simulink model "BaseEngineController_A02_bak"  (Simulink 7.7)
settings: Solver=FixedStepDiscrete  StartTime=0.0  StopTime=10.0  RelTol=1e-3
PreLoadFcn:
  | try, eval([bdroot '_setup']); catch, eval('model_setup', ''); end
InitFcn:
  | try, eval([bdroot '_setup']); catch, eval('model_setup', ''); end
undefined symbols (not defined in the file or in its folder): ECU555, High, motohawk_build_version, motohawk_get_faults, motohawk_trunc_name, motohawk_vardec_config_group, motohawk_vardec_path, True
libraries: MotoHawk_lib x1145, AutoDoc_lib x786, motohawk_control_primitives_lib x114, motohawk_engine_lib x19, motohawk_annotations_lib x12, simulink x11, simulink_extras x1
(part 1 of 23)
Description:
  | MI07 SECM48
CloseFcn:
  | rtwprivate ssgencode ModelCloseRequest BaseEngineController_A02
settings Model: MotoHawkBuildVersion=116  MotoHawkBuildStats=struct('TotalFLASH',{373552},'TotalEEPROM',{1254},'TotalRAM',{26040},'AppFLASH',{154160},'AppEEPROM',{1080},'AppRAM',{7766},'HeapRAM',{4096},'BuildSuccessCount',{8},'BuildErrorCount',{29},'OSRAM',{4962},'StackRAM',{9216},'FgndTimeStackSize',{4096},'FgndAngleStackSize',{1024},'BgndStackSize',{2048},'IdleStackSize',{1024},'InterruptStackSize',{1024},'RTWBlockIOSize',{2192},'RTWDWorkSize',{1776},'RTWPrevZCSize',{0},'VolatileSize',{1668},'NonVolatileSize',{1080},'ConstantSize',{18616},'NonVolatileFormatID',{32179})  MotoHawkModelSavedName=BaseEngineController_A02_bak  MotoHawkModelGUID=dbd4ef7b-9d85-4e51-bb-d0-da-ee-71-31-1c  MotoHawkModelOpenTime=2018 4 5 16 53 42.812  MotoHawkModelElapsedTime=-3947112212.687  MotoHawkBuildGUID=66e2ce16-351c-4ae6-a6-cb-00-88-ae-da-ff  MotoHawkBuildInfo=struct('model_name',{'BaseEngineController_A02'},'motohawk_version',{'2011a_sp0.184'},'motohawk_component_version',{'0.0.7'},'matlab_version',{'7.12.0'},'build_version',{'115'},'module_type',{'ECU555-80'},'layout_type',{'DEV'},'processor_family',{'PPC'},'processor_type',{'MPC555'},'toolchain_vendor',{'gcc-powerpc-eabi'},'toolchain_version',{'4.4.0'},'component',{0},'archive_file',{'PCM.a'},'enable_build',{1},'fptype',{0},'flash_size',{368640},'ee_size',{8192},'ram_size',{26624},'decram_size',{0},'module_memory_def',{'MPC555_ModMemoryDef_8KEE_Dev'},'eri_table0_rev',{9},'max_eri_table_size',{65535},'use_debug_vardecs',{1},'use_info_vardecs',{1},'fgnd_base_period_ms',{5},'bgnd_base_period_ms',{50},'root_build_dir',{'C:\Users\HYBRID\Documents\GitHub\Wisconsin-Hybrid\EngineController_Rev1'},'build_dir',{'C:\Users\HYBRID\Documents\GitHub\Wisconsin-Hybrid\EngineController_Rev1\BaseEngineController_A02_Build'},'dll_name',{'BaseEngin_115'},'srz_name',{'BaseEngineController_A02_115'},'fgnd_stack_size',{4096},'fgnd_angle_stack_size',{1024},'bgnd_stack_size',{2048},'idle_stack_size',{1024},'irq_stack_size',{1024},'heap_size',{4096},'heap_margin',{409},'install_dll_dir',{'C:\ECUFiles\TDBDLL\'},'install_srz_dir',{'C:\ECUFiles\Programs'},'app_int_stack_size',{1024},'shutdown_stack_size',{1024},'mingw_dir',{'C:\PROGRA~2\Woodward\DEVELO~1\TOOLCH~1\GCC\win32-pe\4_4_0\'},'motohawk_dir',{'C:\PROGRA~2\Woodward\MCS\MotoHawk\2011A_~1.184'},'toolchain_dir',{'C:\Program Files (x86)\Woodward\DevelopmentTools\Toolchains\GCC\powerpc-eabi\4_4_0\'},'matlab_root',{'C:\Program Files (x86)\MATLAB\R2011a'},'model_guid',{'34a85f28-21b7-4112-82-b0-87-11-cc-cb-8f'},'build_guid',{'66e2ce16-351c-4ae6-a6-cb-00-88-ae-da-ff'},'token_guid',{'DD8AD11F95A6FC42BFF9AC780840E4B4'})  RegisteredStructs=struct('Name',{'FreezeFrame','FreezeFrames'},'Data',{struct('Name',{int32(0)},'Code',{int32(0)},'Var1',{int32(0)},'Var2',{int32(0)},'Var3',{int32(0)},'Var4',{int32(0)},'Var5',{int32(0)},'Var6',{int32(0)},'Var7',{int32(0)},'Var8',{int32(0)},'Var9',{int32(0)},'Var10',{int32(0)}),struct('Name',{int32(0)},'Code',{int32(0)},'Var1',{int32(0)},'Var2',{int32(0)},'Var3',{int32(0)},'Var4',{int32(0)},'Var5',{int32(0)},'Var6',{int32(0)},'Var7',{int32(0)},'Var8',{int32(0)},'Var9',{int32(0)},'Var10',{int32(0)})},'Enums',{{{'None','APP_Sensors_Conflict','CamEdgesFault','CamSyncFault','CrankEdgesFault','CrankSyncFault','ECTOverTempFault','ECTRangeHigh','ECTRangeLow','ECT_IR_Fault','EST1_Open','EST1_Short','EST2_Open','EST2_Short','EST3_Open','EST3_Short','EST4_Open','EST4_Short','EST5_Open','EST5_Short','EST6_Open','EST6_Short','EST7_Open','EST7_Short','EST8_Open','EST8_Short','ETCSpringTest','ETC_Open_Fault','ETC_Sticking','FuelAdaptRangeHi','FuelAdaptRangeLow','FuelTempRangeHigh','FuelTempRangeLow','HBridgeFault_ETC','HardOverspeed','IATRangeHigh','IATRangeLow','IAT_IR_Fault','Inj1Open','Inj2Open','Inj3Open','Inj4Open','Inj5Open','Inj6Open','Inj7Open','Inj8Open','LSDFault_FuelPump','LSDFault_MIL','LSOFault_VVT','MAFRangeHigh','MAFRangeLow','MAPRangeHigh','MAPRangeLow','MAPTimeRangeHigh','MAPTimeRangeLow','MAP_IR_HI','MAP_IR_LO','MAP_STICKING','MediumOverspeed','O2FailedLean','O2FailedRich','O2NotActive','OilTempRangeHigh','OilTempRangeLow','SensVoltRangeHigh','SensVoltRangeLow','SoftOverspeed','SysVoltRangeHigh','SysVoltRangeLow','TPS_Sensors_Conflict'}},{{'None','CamEdgesFault','CamSyncFault','CrankEdgesFault','CrankSyncFault','ECTOverTempFault','ECTRangeHigh','ECTRangeLow','ECTSevereOverTempFault','EGRSenseRangeHigh','EGRSenseRangeLow','EST1_Open','EST1_Short','EST2_Open','EST2_Short','ETCSpringTest','ETC_Open_Fault','ETC_Sticking','FuelAdaptRangeHi','FuelAdaptRangeLow','FuelPresHigh_Fault','FuelPresLow_Fault','FuelPresRangeHigh','FuelPresRangeLow','FuelSensRangeHigh','FuelSensRangeLow','HBridgeFault_ETC','HardOverspeed','IATRangeHigh','IATRangeLow','Inj1Open','Inj2Open','LSDFault_FuelPump','LSDFault_OilPump','LSDFault_OxygenHeater','MAFRangeHigh','MAFRangeLow','MAPRangeHigh','MAPRangeLow','MAPTimeRangeHigh','MAPTimeRangeLow','MAP_IR_HI','MAP_IR_LO','MAP_STICKING','MediumOverspeed','NoOil_Fault','O2FailedLean','O2FailedRich','O2NotActive','O2_PostCatRangeHigh','O2_PostCatRangeLow','OilPresRangeHigh','OilPresRangeLow','SensVoltRangeHigh','SensVoltRangeLow','SoftOverspeed','SysVoltLow_Fault','SysVoltRangeHigh','SysVoltRangeLow','TPS1AdaptHiMax','TPS1AdaptHiMin','TPS1AdaptLoMax','TPS1AdaptLoMin','TPS1RangeHigh','TPS1RangeLow','TPS2AdaptHiMax','TPS2AdaptHiMin','TPS2AdaptLoMax','TPS2AdaptLoMin','TPS2RangeHigh','TPS2RangeLow','TPS_Sensors_Conflict'}}})  Creator=dbocci  LastModifiedBy=HYBRID
settings OptimizationCC: InlineParams=on  InitFltsAndDblsToZero=on  OptimizeModelRefInitCode=on  LifeSpan=1
settings DebuggingCC: SolverPrmCheckMsg=none  InheritedTsInSrcMsg=none  TasksWithSamePriorityMsg=none  StrictBusMsg=Warning
settings HardwareCC: ProdEndianess=BigEndian  ProdHWDeviceType=Specified
settings RTWCC: SystemTargetFile=motohawk_ert_rtw.tlc  TLCOptions="-aCustomNonFinites=\"motohawk_genrtnonfinite.tlc\""  MakeCommand=motohawk_make_rtw  TemplateMakefile=motohawk_ert_rtw.tmf  Description=MotoHawk(tm) Rapid Prototyping  ProcessScript=motohawk_ert_rtw_make_rtw_hook
settings CodeAppCC: IgnoreCustomStorageClasses=off  MaxIdLength=100  CustomSymbolStrField=$H$N$M
settings STFCustomTargetCC: FunctionExecutionProfile=off  ModelReferenceCompliant=off  CombineOutputUpdateFcns=on  ERTCustomFileBanners=on  SupportContinuousTime=off  SupportNonInlinedSFcns=off  SystemTargetFile=motohawk_ert_rtw.tlc
settings ERTTargetCC: FunctionExecutionProfile=off  MultiwordLength=2048  ModelReferenceCompliant=off  ParMdlRefBuildCompliant=off  CompOptLevelCompliant=off  ERTFirstTimeCompliant=off  ModelStepFunctionPrototypeControlCompliant=off  CPPClassGenCompliant=off  ERTCustomFileTemplate=example_file_process.tlc  ConvertIfToSwitch=off

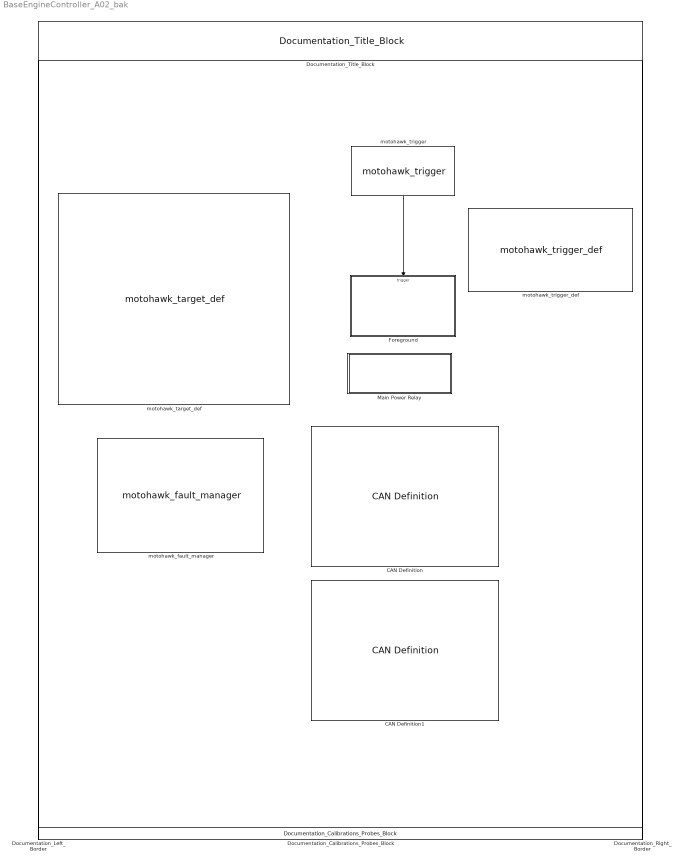
== system BaseEngineController_A02_bak ==
  CAN Definition : MotoHawk_lib/CAN Blocks/CAN Definition  Priority=-100  nam=['CAN_' num2str(1)]  text_resource=CAN1  code_resource=NONE  val_resource=-1  target=ECU555-80  bus=-2  btime=500 kbaud  pre=1  prop=0  pseg1=0  pseg2=0  resyn=0  tx_queue_size=16  rx_queue_size=16  use_protocol=on  city_id=hex2dec('B')  access_level=4  mototune_group='System | System Config | Communication Config'
  CAN Definition1 : MotoHawk_lib/CAN Blocks/CAN Definition  Priority=-100  nam=['CAN_' num2str(2)]  text_resource=CAN2  code_resource=NONE  val_resource=-1  target=ECU555-80  bus=-2  btime=500 kbaud  pre=1  prop=0  pseg1=0  pseg2=0  resyn=0  tx_queue_size=16  rx_queue_size=16  use_protocol=on  city_id=hex2dec('B')  access_level=4  mototune_group='System | System Config | Communication Config'
  Documentation_Calibrations_Probes_Block : AutoDoc_lib/Documentation_Calibrations_Probes_Block
  Documentation_Left_Border : AutoDoc_lib/Documentation_Left_Border  layout=Portrait
  Documentation_Right_Border : AutoDoc_lib/Documentation_Right_Border  layout=Portrait
  Documentation_Title_Block : AutoDoc_lib/Documentation_Title_Block
  Foreground : Subsystem  TreatAsAtomicUnit=on
      settings: RTWSystemCode=Function  RTWFcnNameOpts=Use subsystem name  RTWFileNameOpts=Use subsystem name
  Main Power Relay : Subsystem  AncestorBlock=MotoHawk_lib/Extra Development Blocks/Main Power Relay  mask(MotoHawk Main Power Relay Control){on_delay_count=2, off_delay_count=5, group='System | Main Power Relay'}
      mask prompts: on_delay_count "On Delay Count"; off_delay_count "Off Delay Count"; group "MotoTune Group"
      mask help:
      | This block provides basic control of the main power relay, by following the ECUP voltage input pin.
      |
      | Regardless of where the block is placed in a design, the strategy will execute every 50ms with background priority.
      |
      | Note that this block is simply composed of other primitive MotoHawk blocks and may be used as a starting point for a more complicated strategy.
      |
      | On Delay:  Once the ECU power goes high, after this period, the Main Power Relay will be turned on.
      | Off Delay:  Once the ECU power goes low, after this period, the Main Power Relay will be turned off.
      |
      | Note that one tick before MPRD is turned off, non-volatile variables will be stored, and the Shutdown event will be posted.  It is not necessary to use the Shutdown event.
      |
      | To delay shutdown based on Foreground events, use a data storage write block 'ShutDownTasksComplete' set as a boolean in the Foreground controller to initiate actual shutdown following completion of the tasks.
      |
      | ---------------------------------------------------------------------
      | Copyright 2005 MotoTron, Inc.  All Rights Reserved.
      mask init:
      | on_delay_lbl = sprintf('\nOn Delay: %d ms', on_delay_count * 50);
      | off_delay_lbl = sprintf('\nOff Delay: %d ms', off_delay_count * 50);
      mask Help: eval('motohawk_block_help(get_param(gcb,''MaskType''))');
      mask Display:
      | patch([0 0 1 1], [0 1 1 0], [220/255 220/255 220/255])
      | patch([0 0 1 1], [0 0.075 0.075 0], [230/255 0/255 0/255])
      | patch([0 0 1 1], [0.025 0.05 0.05 0.025], [150/255 0/255 0/255])
      | patch([0 0 1 1], [0 0.025 0.025 0], [0/255 0/255 0/255])
      | 
      | disp(['Main Power Relay' on_delay_lbl off_delay_lbl]);
  motohawk_fault_manager : MotoHawk_lib/Fault Management Blocks/motohawk_fault_manager  storage=FLASH  read_level=1  write_level=1  clear_level=1  xytype=uint16  mototune_group='Diagnostics & Protection | Faults'
  motohawk_target_def : MotoHawk_lib/motohawk_target_def  Priority=-65535  target=ECU555-80  layout=DEV  fptype=single (32 bits)  fgnd_stack_size=4*1024  fgnd_angle_stack_size=1*1024  bgnd_stack_size=2*1024  idle_stack_size=1*1024  irq_stack_size=1*1024  app_int_stack_size=1024  shutdown_stack_size=1024  heap_size=4*1024  show_margins=off  fgnd_stack_margin=(3*1024) * 0.1  fgnd_angle_stack_margin=0  bgnd_stack_margin=(2*1024) * 0.1  idle_stack_margin=(1024) * 0.1  irq_stack_margin=(1536) * 0.1  app_int_stack_margin=(1024) * 0.1  shutdown_stack_margin=(1024) * 0.1  heap_margin=(4*1024) * 0.1  comp_name=bdroot  comp_img='mh.jpg'  comp_security=No Encryption  comp_build_pass='mhbuild'  comp_sim_pass='mhsim'  comp_multi=off  show_filenames=on  dll_name=motohawk_trunc_name(bdroot, ['_' motohawk_build_version], 13)  srz_name=motohawk_trunc_name(bdroot, ['_' motohawk_build_version], 50)  build_dir=''  comp_dir='.'
  motohawk_trigger : MotoHawk_lib/Trigger Blocks/motohawk_trigger  Tag=Trigger  Priority=0  trigger=FGND_RTI_PERIODIC  function_name=Trigger_FGND_RTI_PERIODIC_10578p0002  priority_order=0  inherited=off  run_disabled=off  override_app_monitor=off  target=ECU555_80
  motohawk_trigger_def : MotoHawk_lib/Trigger Blocks/motohawk_trigger_def  fgnd_rti_rate=5000  bgnd_base_multiple=10  read_level=1  write_level=1  mototune_group='System | System Config | Trigger Config'
  wires:
  motohawk_trigger/1 -> Foreground/Trigger

== system BaseEngineController_A02_bak/Foreground ==
  f : TriggerPort  TriggerType=function-call
  Control : Subsystem  mask(Display Image File){image_file='pcm555.jpg'}
      mask prompts: image_file "Image File"
      mask help:
      | Enter an image file on the current MATLAB path to display on this block.
      |
      | Double-clicking this block opens the contents of the subsystem.  Right-click and select 'Mask parameters...' to select a new image file.
      |
      | To use just the mask of this block in a model, but supply your own contents, break the link to the library.
      mask init:
      | try,
      | 	img = imread(image_file);
      | 	motohawk_block_image(gcb, image_file);
      | catch,
      | 	img = imread('image_not_found.jpg');
      | end
      settings: OpenFcn=open_system(gcb,'force')  MoveFcn=motohawk_block_image(gcb, motohawk_get_param_ws(gcb, 'image_file'));
      mask Display: image(img);
  Documentation_Calibrations_Probes_Block : AutoDoc_lib/Documentation_Calibrations_Probes_Block
  Documentation_Left_Border : AutoDoc_lib/Documentation_Left_Border  layout=Portrait
  Documentation_Right_Border : AutoDoc_lib/Documentation_Right_Border  layout=Portrait
  Documentation_Title_Block : AutoDoc_lib/Documentation_Title_Block
  Inputs : Subsystem  mask(Display Image File){image_file='sensor.jpg'}
      mask prompts: image_file "Image File"
      mask help:
      | Enter an image file on the current MATLAB path to display on this block.
      |
      | Double-clicking this block opens the contents of the subsystem.  Right-click and select 'Mask parameters...' to select a new image file.
      |
      | To use just the mask of this block in a model, but supply your own contents, break the link to the library.
      mask init:
      | try,
      | 	img = imread(image_file);
      | 	motohawk_block_image(gcb, image_file);
      | catch,
      | 	img = imread('image_not_found.jpg');
      | end
      settings: OpenFcn=open_system(gcb,'force')  MoveFcn=motohawk_block_image(gcb, motohawk_get_param_ws(gcb, 'image_file'));
      mask Display: image(img);
  Outputs : Subsystem  mask(Display Image File){image_file='actuator.jpg'}
      mask prompts: image_file "Image File"
      mask help:
      | Enter an image file on the current MATLAB path to display on this block.
      |
      | Double-clicking this block opens the contents of the subsystem.  Right-click and select 'Mask parameters...' to select a new image file.
      |
      | To use just the mask of this block in a model, but supply your own contents, break the link to the library.
      mask init:
      | try,
      | 	img = imread(image_file);
      | 	motohawk_block_image(gcb, image_file);
      | catch,
      | 	img = imread('image_not_found.jpg');
      | end
      settings: OpenFcn=open_system(gcb,'force')  MoveFcn=motohawk_block_image(gcb, motohawk_get_param_ws(gcb, 'image_file'));
      mask Display: image(img);
  wires:
  Control/1(Actuators) -> Outputs/1(Actuators)
  Inputs/1(SensorBus) -> Control/1(Sensors)

== system BaseEngineController_A02_bak/Main Power Relay ==
  note: The saving and restoring of non-volatile variables
      | must be called from a background priority task.
  note: Always execute Main Power Relay control in the background.
  Documentation_Calibrations_Probes_Block : AutoDoc_lib/Documentation_Calibrations_Probes_Block
  Documentation_Left_Border : AutoDoc_lib/Documentation_Left_Border  layout=Portrait
  Documentation_Right_Border : AutoDoc_lib/Documentation_Right_Border  layout=Portrait
  Documentation_Title_Block : AutoDoc_lib/Documentation_Title_Block
  Main Power Relay : Subsystem  TreatAsAtomicUnit=on
  motohawk_trigger : MotoHawk_lib/Trigger Blocks/motohawk_trigger  Tag=Trigger  Priority=255  trigger=BGND_BASE_PERIODIC  function_name=Trigger_BGND_BASE_PERIODIC_9474p0004  priority_order=255  inherited=on  run_disabled=on  override_app_monitor=off  target=ECU555_80
  wires:
  motohawk_trigger/1 -> Main Power Relay/Trigger

== system BaseEngineController_A02_bak/Foreground/Control ==
  Sensors : Inport
  ActuatorCharacterization : Subsystem
  Controller : Subsystem
  Diagnostics : Subsystem  TreatAsAtomicUnit=on
  Documentation_Calibrations_Probes_Block : AutoDoc_lib/Documentation_Calibrations_Probes_Block
  Documentation_Left_Border : AutoDoc_lib/Documentation_Left_Border  layout=Portrait
  Documentation_Right_Border : AutoDoc_lib/Documentation_Right_Border  layout=Portrait
  Documentation_Title_Block : AutoDoc_lib/Documentation_Title_Block
  VirtualSensors : Subsystem
      settings: RTWSystemCode=Function  RTWFcnNameOpts=Use subsystem name  RTWFileNameOpts=Use function name
  motohawk_function_collector : MotoHawk_lib/Extra Development Blocks/motohawk_function_collector  SystemSampleTime=-1  count=5
  Actuators : Outport
  wires:
  Sensors/1 -> VirtualSensors/1(Raw)
  VirtualSensors/1(Sensors) -> Controller/1(Sensors), ActuatorCharacterization/2(Sensors), Diagnostics/2(Sensors)
  ActuatorCharacterization/1(Actuators) -> Actuators/1
  Controller/1(Actuators) -> ActuatorCharacterization/1(Control), Diagnostics/1(Control)
  Diagnostics/1(Diagnostics) -> ActuatorCharacterization/3(Diagnsotics)
  motohawk_function_collector/1 -> Diagnostics/Trigger

== system BaseEngineController_A02_bak/Foreground/Inputs ==
  Bus Creator : BusCreator  Inputs=7
  CAN Inputs : Subsystem
  Digital : Subsystem
  Documentation_Calibrations_Probes_Block : AutoDoc_lib/Documentation_Calibrations_Probes_Block
  Documentation_Left_Border : AutoDoc_lib/Documentation_Left_Border  layout=Portrait
  Documentation_Right_Border : AutoDoc_lib/Documentation_Right_Border  layout=Portrait
  Documentation_Title_Block : AutoDoc_lib/Documentation_Title_Block
  EngineSpeed : Subsystem
  LinearSensors : Subsystem
  NonLinearSensors : Subsystem
  PotentiometerSensors : Subsystem
  SensorBus : Outport
  wires:
  Digital/1(SensorBus) -> Bus Creator/6
  PotentiometerSensors/1(SensorBus) -> Bus Creator/5
  NonLinearSensors/1(SensorBus) -> Bus Creator/4
  LinearSensors/1(SensorBus) -> Bus Creator/3
  EngineSpeed/2(RPM) -> Bus Creator/2  "RPM"
  EngineSpeed/1(RPMInst) -> Bus Creator/1  "RPMInst"
  Bus Creator/1 -> SensorBus/1
  CAN Inputs/1(Out1) -> Bus Creator/7  "CAN Inputs"

== system BaseEngineController_A02_bak/Foreground/Outputs ==
  Actuators : Inport
  Bus Selector1 : BusSelector  OutputSignals=Controller.FUELP,Sensors.Raw.Digital.ESTOP
  Bus Selector2 : BusSelector  OutputSignals=Controller.OILP
  Bus Selector3 : BusSelector  OutputSignals=ActuatorCharacterization.ETC,Sensors.RawIndexes.SysVolt09Idx,Sensors.Raw.Digital.ESTOP,Sensors.RawProcessed.TimeSinceStall
  Bus Selector4 : BusSelector  OutputSignals=Controller.SOI,ActuatorCharacterization.InjMPW,ActuatorCharacterization.InjEPWOffset,ActuatorCharacterization.FuelCutSeq,Controller.MakeUpEOI
  Bus Selector5 : BusSelector  OutputSignals=ActuatorCharacterization.SparkEnable,ActuatorCharacterization.SparkStartAngle,Controller.SparkAdv,ActuatorCharacterization.SparkCutSeq
  Bus Selector6 : BusSelector  OutputSignals=Controller.O2 Heater
  CAN Out : Subsystem
  Coil Control : Subsystem  TreatAsAtomicUnit=on
  Documentation_Calibrations_Probes_Block : AutoDoc_lib/Documentation_Calibrations_Probes_Block
  Documentation_Left_Border : AutoDoc_lib/Documentation_Left_Border  layout=Portrait
  Documentation_Right_Border : AutoDoc_lib/Documentation_Right_Border  layout=Portrait
  Documentation_Title_Block : AutoDoc_lib/Documentation_Title_Block
  EGR Out : Subsystem
  FuelPump : Subsystem
  HBridge : Subsystem
  Injector Control : Subsystem
  Logical Operator4 : Logic  Operator=NOT  AllPortsSameDT=off  OutDataTypeStr=boolean
  Logical Operator5 : Logic  AllPortsSameDT=off  OutDataTypeStr=boolean
  Logical Operator9 : Logic  Operator=OR  AllPortsSameDT=off  OutDataTypeStr=boolean
  OilPump : Subsystem
  Oxygen Heater : Subsystem
  Relational Operator3 : RelationalOperator  Operator=>  ZeroCross=off  InputSameDT=on
  motohawk_calibration3 : MotoHawk_lib/Calibration & Probing Blocks/motohawk_calibration  nam='ETC_CutPower_StallTime'  val=15  storage=Calibration  show_more=on  name_source=Use Parameter  data_type=Inherit from 'Default Value'  read_level=1  write_level=1  ovr_cals=on  view_as=Number  enum_struct={'Disabled', 'Enabled'}  vector_style=Wide Row  use_help_units=on  help='Cut power to ETC after stalled this long'  units='s'  use_header_enum=off  row_header_enum={}  col_header_enum={}  use_min_max=off  min_val=0  max_val=100  use_precision=on  precision='2.3'  gain=1  offset=0  exp=1  use_mototune_group=on  mototune_group=motohawk_vardec_path(gcb)
  motohawk_trigger : MotoHawk_lib/Trigger Blocks/motohawk_trigger  Tag=Trigger  trigger=FGND_5XRTI_PERIODIC  function_name=Trigger_FGND_5XRTI_PERIODIC_9202p0004  priority_order=1  inherited=on  run_disabled=off  override_app_monitor=off  target=ECU555_80
  wires:
  Bus Selector3/2 -> HBridge/2(SysVoltIdx)  "<SysVolt09Idx>"
  Bus Selector4/5 -> Injector Control/5(MakeUpEOI)  "<MakeUpEOI>"
  Bus Selector3/1 -> HBridge/1(ETCDuty Cycle)  "<ETC>"
  Bus Selector5/4 -> Coil Control/4(CutSequence)  "<SparkCutSeq>"
  Bus Selector4/4 -> Injector Control/4(CutSequence)  "<FuelCutSeq>"
  Bus Selector5/3 -> Coil Control/3(SparkAdvance)  "<SparkAdv>"
  Bus Selector5/2 -> Coil Control/2(SparkStartAngle)  "<SparkStartAngle>"
  Bus Selector4/3 -> Injector Control/3(InjEPWOffset)  "<InjEPWOffset>"
  Bus Selector4/2 -> Injector Control/2(InjMPW)  "<InjMPW>"
  Bus Selector5/1 -> Coil Control/1(SparkEnable)  "<SparkEnable>"
  Bus Selector4/1 -> Injector Control/1(SOI)  "<SOI>"
  Actuators/1 -> Bus Selector3/1, Bus Selector1/1, Bus Selector2/1, EGR Out/1(EGR), CAN Out/1(In1), Bus Selector6/1, Bus Selector5/1, Bus Selector4/1
  motohawk_trigger/1 -> Coil Control/Trigger
  Bus Selector1/2 -> Logical Operator4/1  "<ESTOP>"
  Logical Operator4/1 -> Logical Operator5/2
  Bus Selector1/1 -> Logical Operator5/1  "<FUELP>"
  Logical Operator5/1 -> FuelPump/1(State)
  Logical Operator9/1 -> HBridge/3(ETC_CutPower)
  Bus Selector3/3 -> Logical Operator9/1  "<ESTOP>"
  Relational Operator3/1 -> Logical Operator9/2
  Bus Selector3/4 -> Relational Operator3/1  "<TimeSinceStall>"
  motohawk_calibration3/1 -> Relational Operator3/2
  Bus Selector2/1 -> OilPump/1(State)  "<OILP>"
  Bus Selector6/1 -> Oxygen Heater/1(State)  "<O2 Heater>"

== system BaseEngineController_A02_bak/Main Power Relay/Main Power Relay ==
  note: Provide Data Store
      | Access to MPRD State
  note: Control Main Power Relay
  note: NonVolatile Memory Store/Restore
      | Hooks from MotoTune
  note: Software Reset
      | Hook from MotoTune
  note: When ProcessorReboot goes true, it will force a falling edge to perform a shutdown, save NV vars, shutdown power, and then cause a processor reset if still true
  note: After a delay, turn on or off Main Power Relay
  f : TriggerPort  TriggerType=function-call  SampleTime=-1  ZeroCross=off
  Display Variable Event Boolean : MotoHawk_lib/Extra Development Blocks/Display Variable Event Boolean  SystemSampleTime=-1  nam='ProcessorReboot'  level=1  enum_str={'Run', 'Reboot'}  help_str='Reboots the processor when set.'  group=group
  Display Variable Event Function-Call : MotoHawk_lib/Extra Development Blocks/Display Variable Event Function-Call  SystemSampleTime=-1  nam='RestoreNVFactoryDefaults'  level=1  enum_str={'Run', 'Restore To Defaults'}  help_str='Restores NonVolatile Memory to Factory Defaults'  group='System | NonVolatile Storage'
  Display Variable Event Function-Call1 : MotoHawk_lib/Extra Development Blocks/Display Variable Event Function-Call  SystemSampleTime=-1  nam='StoreNV'  level=1  enum_str={'Run', 'Store To EEPROM'}  help_str='Stores RAM-Shadowed memory to serial EEPROM'  group='System | NonVolatile Storage'
  Documentation_Calibrations_Probes_Block : AutoDoc_lib/Documentation_Calibrations_Probes_Block
  Documentation_Left_Border : AutoDoc_lib/Documentation_Left_Border  layout=Portrait
  Documentation_Right_Border : AutoDoc_lib/Documentation_Right_Border  layout=Portrait
  Documentation_Title_Block : AutoDoc_lib/Documentation_Title_Block
  ECUP Latch : Stateflow chart  TreatAsAtomicUnit=on
  Forced Shutdown Process : Subsystem
  Logical Operator : Logic
  Logical Operator1 : Logic  Operator=NOT
  On Off Delay : Subsystem
  Relational Operator : RelationalOperator  ZeroCross=off  InputSameDT=on
  ShutDown Processes : Subsystem
  Unit Delay3 : UnitDelay  SampleTime=-1
  Unit Delay4 : UnitDelay  SampleTime=-1
  Unit Delay5 : UnitDelay  SampleTime=-1
  motohawk_ain5 : MotoHawk_lib/Analog I//O Blocks/motohawk_ain  res_name=[]  text_resource=ECUP  code_resource=RES_ECUP  val_resource=-1  target=ECU555-80  resource_enum_str=(None)=-1,ECUP=53,DRVP=54,XDRP=55,AN1M=56,AN2M=57,AN3M=58,AN4M=59,AN5M=60,AN6M=61,AN7M=62,AN8M=63,AN9M=64,AN10M=65,AN11M=66,AN12M=67,AN13M=68,AN14M=69,AN15M=70,AN16M=71,AN17M=72,AN18M=73,AN19M=74,O2A=90,O2B=91,XDRP_B=92  altref=5V Reference  typ=uint16  num_adc_bits=10  use_stat=off  use_vardec=off  nam='AIN'  read_level=1  write_level=1  group='System | I/O Pins | Inputs Config'
  motohawk_calibration : MotoHawk_lib/Calibration & Probing Blocks/motohawk_calibration  nam='ECUP_Threshold'  val=100  storage=Calibration  show_more=on  name_source=Use Parameter  data_type=Inherit via back propagation  read_level=1  write_level=1  ovr_cals=on  view_as=Number  enum_struct={'Disabled', 'Enabled'}  vector_style=Wide Row  use_help_units=off  help='ADC voltage threshold above which ECU is considered powered'  units='ADC_counts'  use_header_enum=off  row_header_enum={}  col_header_enum={}  use_min_max=on  min_val=0  max_val=1023  use_precision=off  precision=''  gain=1  offset=0  exp=1  use_mototune_group=on  mototune_group=motohawk_vardec_config_group(group)
  motohawk_data_def : MotoHawk_lib/Data Storage Blocks/motohawk_data_def  nam='MPRD'  container_nam=''  data=false  typ=Inherit from 'Initial Value'  struct_nam='MyStruct'  storage=Volatile  outputRef=off  use_vardec=on  window=Display  read_level=1  write_level=(Read Only)  ovr_cals=on  view_as=Enumeration  enum_struct={'Disabled', 'Enabled'}  vector_style=Wide Row  use_help_units=on  help='Indicates whether the main power relay is active'  units=''  use_header_enum=off  row_header_enum={}  col_header_enum={}  use_min_max=off  min_val=-inf  max_val=inf  use_precision=off  precision=''  gain=1  offset=0  exp=1  use_mototune_group=on  mototune_group=group  eval_name=MPRD  data_stores=motohawk_sfun_data_def_data_stores  struct_instances=motohawk_sfun_data_def_struct_instances  vardecs=motohawk_sfun_data_def_vardecs
  motohawk_data_def1 : MotoHawk_lib/Data Storage Blocks/motohawk_data_def  nam='ShutDownTasksComplete'  container_nam=''  data=false  typ=boolean  struct_nam='MyStruct'  storage=Volatile  outputRef=off  use_vardec=on  window=Display  read_level=1  write_level=1  ovr_cals=on  view_as=Enumeration  enum_struct={'False', 'True'}  vector_style=Wide Row  use_help_units=off  help=''  units=''  use_header_enum=off  row_header_enum={}  col_header_enum={}  use_min_max=off  min_val=-inf  max_val=inf  use_precision=off  precision=''  gain=1  offset=0  exp=1  use_mototune_group=on  mototune_group='System | Main Power Relay'  eval_name=ShutDownTasksComplete  data_stores=motohawk_sfun_data_def_data_stores  struct_instances=motohawk_sfun_data_def_struct_instances  vardecs=motohawk_sfun_data_def_vardecs
  motohawk_data_read : MotoHawk_lib/Data Storage Blocks/motohawk_data_read  source=Lookup By Name  structure=Scalar  operation=Read entire data structure at once  sz_vec=4  sz_row=2  sz_col=3  typ=boolean  nam='ShutDownTasksComplete'  checkNull=on  struct_inst_name='Struct1'  struct_nam='MyStruct'
  motohawk_data_write : MotoHawk_lib/Data Storage Blocks/motohawk_data_write  source=Lookup By Name  structure=Scalar  operation=Write entire data structure at once  sz_vec=-1  sz_row=3  sz_col=2  typ=boolean  nam='MPRD'  checkNull=off  write_read_only=off  struct_inst_name='Struct1'  struct_name='MyStruct'
  motohawk_dout : MotoHawk_lib/Digital I//O Blocks/motohawk_dout  resource_name=[]  text_resource=MPRD  target=ECU555-80  use_diag=off  use_vardec=off  nam='DOUT'  read_level=1  write_level=1  group='System | I/O Pins | Outputs Config'
  motohawk_probe : MotoHawk_lib/Calibration & Probing Blocks/motohawk_probe  nam='ECUP_Enabled'  show_more=on  name_source=Use Parameter  read_level=1  view_as=Enumeration  enum_struct={'Disabled', 'Enabled'}  vector_style=Wide Row  use_help_units=off  help=''  units=''  use_header_enum=off  row_header_enum={}  col_header_enum={}  use_precision=off  precision=''  gain=1  offset=0  exp=1  use_mototune_group=on  mototune_group=group
  motohawk_restore_nvmem : MotoHawk_lib/Data Storage Blocks/motohawk_restore_nvmem  SystemSampleTime=-1
  motohawk_store_nvmem : MotoHawk_lib/Data Storage Blocks/motohawk_store_nvmem  SystemSampleTime=-1
  wires:
  Logical Operator/1 -> ShutDown Processes/2(Input State)
  ShutDown Processes/1(MPRD State) -> motohawk_data_write/1, motohawk_dout/1
  motohawk_data_read/1 -> ECUP Latch/2(ShutDownTasks)
  Relational Operator/1 -> Forced Shutdown Process/1(ECUP State), motohawk_probe/1, ECUP Latch/1(ECUP)
  ECUP Latch/1(MPRDState) -> On Off Delay/1(In)
  Unit Delay5/1 -> ShutDown Processes/1(Manual Reset)
  Unit Delay4/1 -> Unit Delay5/1
  Unit Delay3/1 -> Unit Delay4/1
  Display Variable Event Function-Call1/1 -> motohawk_store_nvmem/Trigger
  Display Variable Event Function-Call/1 -> motohawk_restore_nvmem/Trigger
  Logical Operator1/1 -> Logical Operator/1
  On Off Delay/1(Out) -> Logical Operator/2
  Display Variable Event Boolean/1 -> Unit Delay3/1, Logical Operator1/1
  motohawk_calibration/1 -> Relational Operator/2
  motohawk_ain5/1 -> Relational Operator/1

== system BaseEngineController_A02_bak/Foreground/Control/ActuatorCharacterization ==
  Control : Inport
  Sensors : Inport  Port=2
  Diagnsotics : Inport  Port=3
  Bus Creator1 : BusCreator  Inputs=9
  Bus Creator3 : BusCreator
  Bus Selector1 : BusSelector  OutputSignals=AirCalcs.Baro,Raw.LinearSensors.MAP_Angular,RawIndexes.SysVolt09Idx,Raw.RPM
  Bus Selector2 : BusSelector  OutputSignals=EGR
  Bus Selector3 : BusSelector  OutputSignals=RawProcessed.TPS
  Bus Selector4 : BusSelector  OutputSignals=Throttle
  Bus Selector5 : BusSelector  OutputSignals=SparkAdv,SparkEnergy,FPC,FuelMult
  Bus Selector6 : BusSelector  OutputSignals=Raw.LinearSensors.EGRPos
  CompareTo : motohawk_control_primitives_lib/CompareTo  SystemSampleTime=-1  op===  val=0
  Documentation_Calibrations_Probes_Block : AutoDoc_lib/Documentation_Calibrations_Probes_Block
  Documentation_Left_Border : AutoDoc_lib/Documentation_Left_Border  layout=Portrait
  Documentation_Right_Border : AutoDoc_lib/Documentation_Right_Border  layout=Portrait
  Documentation_Title_Block : AutoDoc_lib/Documentation_Title_Block
  EGR Characterization : Subsystem
  Electronic Throttle Controller : Subsystem  TreatAsAtomicUnit=on
      mask Display:
      | patch([0 0 1 1], [0 0.075 0.075 0], [230/255 0/255 0/255])
      | patch([0 0 1 1], [0.025 0.05 0.05 0.025], [150/255 0/255 0/255])
      | patch([0 0 1 1], [0 0.025 0.025 0], [0/255 0/255 0/255])
      | 
      | disp('Electronic Throttle');
  Injector Characterization : Subsystem  Description=|
      | /* Name: InjGain Unit:ms/mg ClassID:VAR StorageID:FLASH Access:RW4+RW3+NA2+NA1 TypeID:UINT2 Gain:0.0001       
      |    Min:0.0000 Max:6.5500 Format:5.4 UpdateID:REMOTE Group:"Actuators | Injectors | Config" Help:"Gain valued "
      |    "used in the linear transformation of FPC to mechanical pulsewidth." VardecSig:6C */                       
      |                                                                                                               
      | /* Name: InjOfst Unit:ms ClassID:VAR StorageID:FLASH Access:RW4+RW3+NA2+NA1 TypeID:SINT2 Gain:0.0001          
      |    Min:-3.2768 Max:3.2767 Format:5.4 UpdateID:REMOTE Group:"Actuators | Injectors | Config" Help:"Offset "    
      |    "valued used in the linear transformation of FPC to mechanical pulsewidth." VardecSig:6C */                
      |                                                                                                               
      | /* Name: SysVolt10IdxArr Unit:volt ClassID:ORDARR StorageID:FLASH Access:RW4+RW3+NA2+NA1 TypeID:UINT1         
      |    Gain:0.1 Min:0.00 Max:25.50 Format:4.2 UpdateID:REMOTE OrdLen:10 Group:"Global Index Arrays | Voltage"     
      |    Help:"Ten breakpoint system voltage index array." VardecSig:6C */                                          
      |                                                                                                               
      | /* Name: InjTurnOnTbl Unit:ms ClassID:TBL StorageID:FLASH Access:RW4+RW3+NA2+NA1 TypeID:UINT2 Gain:0.001      
      |    Min:0.0000 Max:65.5350 Format:5.4 UpdateID:REMOTE OrdArr:SysVolt10IdxArr Group:"Actuators | Injectors"     
      |    Help:"Injector turn on time as a function of system voltage." VardecSig:6C */                              
      |                                                                                                               
      | /* Name: InjDeltaPress10IdxArr Unit:kPa ClassID:ORDARR StorageID:FLASH Access:RW4+RW3+NA2+NA1 TypeID:UINT2    
      |    Gain:0.1 Min:0.0 Max:6553.0 Format:5.1 UpdateID:REMOTE OrdLen:10 Group:"Actuators | Injectors" Help:"Ten " 
      |    "breakpoint fuel delta pressure index array." VardecSig:6C */                                              
      |                                                                                                               
      | /* Name: FueldPCompTbl Unit:kPa ClassID:TBL StorageID:FLASH Access:RW4+RW3+NA2+NA1 TypeID:UINT2 Gain:0.1      
      |    Min:0.0 Max:6553.0 Format:5.1 UpdateID:REMOTE OrdArr:InjDeltaPress10IdxArr Group:"Actuators | Injectors"   
      |    Help:"Injector delta pressure compensation table." VardecSig:6C */                                         
      |                                                                                                               
      | /* Name: InjTurnOffTbl Unit:ms ClassID:TBL StorageID:FLASH Access:RW4+RW3+NA2+NA1 TypeID:UINT2                
      |    Gain:0.01 Min:0.00 Max:655.00 Format:5.2 UpdateID:REMOTE OrdArr:InjDeltaPress10IdxArr Group:"Actuators | " 
      |    "Injectors" Help:"Injector turn off time table." VardecSig:6C */                                           
      |                                                                                                               
      | /* Name: InjMPW Unit:ms ClassID:VAR StorageID:RAM Access:RO4+RO3+NA2+NA1 TypeID:UINT2 Gain:0.01 Min:0.00      
      |    Max:655.35 Format:5.2 UpdateID:BACKGND Group:"Actuators | Injectors" Help:"Injector mechanical pulse "     
      |    "width." VardecSig:6C */                                                                                   
      |                                                                                                               
      | /* Name: InjEPW Unit:ms ClassID:VAR StorageID:RAM Access:RO4+RO3+NA2+NA1 TypeID:UINT2 Gain:0.01 Min:0.00      
      |    Max:655.35 Format:5.2 UpdateID:BACKGND Group:"Actuators | Injectors" Help:"Injector electrical pulse "     
      |    "width." VardecSig:6C */                                                                                   
      |                                                                                                               
      | /* Name: InjDeltaPress Unit:kPa ClassID:VAR StorageID:RAM Access:RO4+RO3+NA2+NA1 TypeID:UINT2 Gain:0.01       
      |    Min:0.00 Max:655.35 Format:5.2 UpdateID:BACKGND Group:"Actuators | Injectors" Help:"Delta pressure across "
      |    "the injector." VardecSig:6C */                                                                            
      | /* Name: FuelFlowCompMap Unit:mult ClassID:MAP StorageID:RAM Access:RO4+RO3+NA2+NA1 TypeID:UINT2              
      |    Gain:0.0001 Min:0.0000 Max:2.0000 Format:5.4 UpdateID:BACKGND XOrdArr:IndTorque10IdxArr YOrdArr:RPM10IdxArr
      |    Group:"Actuators | Injectors" Help:"Correction for injector fuel flow differences between injector test "  
      |    "bench and engine operating in the conditions. This map should only be calibrated after volumetric "       
      |    "efficiency has been calibrated to a reference airflow measurement device." VardecSig:6C */
      mask Description: Injector characterization block.
      mask Help: Converts the calculated fuel charge mass (FPC) value to the required electrical pulsewidth. Requires gain, offset, turn-on time vs. voltage, turn-off time vs. injector delta pressure and delta pressure pulsewidth compensation. A choice of five different injector delta pressure calculation methods are offered.
      mask Display:
      | patch([0 0 1 1], [0 0.075 0.075 0], [230/255 0/255 0/255])
      | patch([0 0 1 1], [0.025 0.05 0.05 0.025], [150/255 0/255 0/255])
      | patch([0 0 1 1], [0 0.025 0.025 0], [0/255 0/255 0/255])
      | 
      | disp('INJECTOR\nCHRZN.\n(High Z Only)')
  SequenceCutPattern : Subsystem
      mask Display:
      | patch([0 0 1 1], [0 0.075 0.075 0], [230/255 0/255 0/255])
      | patch([0 0 1 1], [0.025 0.05 0.05 0.025], [150/255 0/255 0/255])
      | patch([0 0 1 1], [0 0.025 0.025 0], [0/255 0/255 0/255])
      | 
      | disp('REV LIMITER\nACTION\n8 CYLINDER')
  Spark Advance to Start Angle : Subsystem  Description=|  TreatAsAtomicUnit=on
      | /* Name: CoilDrvrDlyTime Unit:us ClassID:VAR StorageID:FLASH Access:RW4+RW3+NA2+NA1 TypeID:UINT2 Gain:0.1       
      |    Min:0.00 Max:6553.50 Format:6.2 UpdateID:REMOTE Group:"Actuators | Coils" Help:"Coil driver delay time "     
      |    "between initiation of the EST signal and the initiation of coil dwell." VardecSig:6C */                     
      |                                                                                                                 
      | /* Name: SysVolt10IdxArr Unit:volt ClassID:VAR StorageID:FLASH Access:RW4+RW3+NA2+NA1 TypeID:UINT1 Gain:0.1     
      |    Min:0.00 Max:25.50 Format:4.2 UpdateID:REMOTE Group:"Global Index Arrays | Voltage" Help:"Ten breakpoint "   
      |    "system voltage index array." VardecSig:6C */                                                                
      |                                                                                                                 
      | /* Name: SpkEnergy10IdxArr Unit:volt ClassID:VAR StorageID:FLASH Access:RW4+RW3+NA2+NA1 TypeID:UINT1            
      |    Gain:0.1 Min:0.00 Max:25.50 Format:4.2 UpdateID:REMOTE Group:"Actuators | Coils" Help:"Ten breakpoint spark "
      |    "energy index array." VardecSig:6C */                                                                        
      |                                                                                                                 
      | /* Name: CoilDwellMap Unit:ms ClassID:MAP StorageID:FLASH Access:RW4+RW3+NA2+NA1 TypeID:UINT2 Gain:0.01         
      |    Min:0.00 Max:655.35 Format:4.2 UpdateID:REMOTE XOrdArr:SpkEnergy10IdxArr YOrdArr:SysVolt10IdxArr             
      |    Group:"Actuators | Coils" Help:"Required coil dwell map as a function of system voltage and spark energy."   
      |    VardecSig:6C */
      mask Type: Stop Angle to Start Angle
      mask Description: Stop Angle to Start Angle calculation.  Subtracts the duration of a Pulse Width in the angle domain from a given Stop Angle.
      mask Display:
      | patch([0 0 1 1], [0 0.075 0.075 0], [230/255 0/255 0/255])
      | patch([0 0 1 1], [0.025 0.05 0.05 0.025], [150/255 0/255 0/255])
      | patch([0 0 1 1], [0 0.025 0.025 0], [0/255 0/255 0/255])
  Spark Coil Characterization : Subsystem  Description=|  TreatAsAtomicUnit=on
      | /* Name: CoilDrvrDlyTime Unit:us ClassID:VAR StorageID:FLASH Access:RW4+RW3+NA2+NA1 TypeID:UINT2 Gain:0.1       
      |    Min:0.00 Max:6553.50 Format:6.2 UpdateID:REMOTE Group:"Actuators | Coils" Help:"Coil driver delay time "     
      |    "between initiation of the EST signal and the initiation of coil dwell." VardecSig:6C */                     
      |                                                                                                                 
      | /* Name: SysVolt10IdxArr Unit:volt ClassID:VAR StorageID:FLASH Access:RW4+RW3+NA2+NA1 TypeID:UINT1 Gain:0.1     
      |    Min:0.00 Max:25.50 Format:4.2 UpdateID:REMOTE Group:"Global Index Arrays | Voltage" Help:"Ten breakpoint "   
      |    "system voltage index array." VardecSig:6C */                                                                
      |                                                                                                                 
      | /* Name: SpkEnergy10IdxArr Unit:volt ClassID:VAR StorageID:FLASH Access:RW4+RW3+NA2+NA1 TypeID:UINT1            
      |    Gain:0.1 Min:0.00 Max:25.50 Format:4.2 UpdateID:REMOTE Group:"Actuators | Coils" Help:"Ten breakpoint spark "
      |    "energy index array." VardecSig:6C */                                                                        
      |                                                                                                                 
      | /* Name: CoilDwellMap Unit:ms ClassID:MAP StorageID:FLASH Access:RW4+RW3+NA2+NA1 TypeID:UINT2 Gain:0.01         
      |    Min:0.00 Max:655.35 Format:4.2 UpdateID:REMOTE XOrdArr:SpkEnergy10IdxArr YOrdArr:SysVolt10IdxArr             
      |    Group:"Actuators | Coils" Help:"Required coil dwell map as a function of system voltage and spark energy."   
      |    VardecSig:6C */
      mask Description: Coil characterization block.
      mask Help: Given the desired spark energy and system voltage, this block determines the required coil dwell period. Requires calibration of the dwell map and the delay time.
      mask Display:
      | patch([0 0 1 1], [0 0.075 0.075 0], [230/255 0/255 0/255])
      | patch([0 0 1 1], [0.025 0.05 0.05 0.025], [150/255 0/255 0/255])
      | patch([0 0 1 1], [0 0.025 0.025 0], [0/255 0/255 0/255])
      | 
      | disp('COIL\nCHRZN.')
  motohawk_data_read : MotoHawk_lib/Data Storage Blocks/motohawk_data_read  source=Lookup By Name  structure=Scalar  operation=Read scalar from location by index  sz_vec=4  sz_row=2  sz_col=3  typ=boolean  nam='AirControlSource'  checkNull=on  struct_inst_name='Struct1'  struct_nam='MyStruct'
  motohawk_trigger : MotoHawk_lib/Trigger Blocks/motohawk_trigger  Tag=Trigger  trigger=FGND_5XRTI_PERIODIC  function_name=Trigger_FGND_5XRTI_PERIODIC_1407p0004  priority_order=1  inherited=on  run_disabled=off  override_app_monitor=off  target=ECU555_80
  motohawk_trigger1 : MotoHawk_lib/Trigger Blocks/motohawk_trigger  Tag=Trigger  trigger=FGND_5XRTI_PERIODIC  function_name=Trigger_FGND_5XRTI_PERIODIC_1408p0004  priority_order=1  inherited=on  run_disabled=off  override_app_monitor=off  target=ECU555_80
  Actuators : Outport
  wires:
  Sensors/1 -> Bus Selector1/1, Bus Creator3/3, Bus Selector3/1, Bus Selector6/1  "Sensors"
  Bus Selector3/1 -> Electronic Throttle Controller/1(TPS)  "<TPS>"
  Electronic Throttle Controller/1(ETC) -> Bus Creator1/5  "ETC"
  Bus Selector4/1 -> Electronic Throttle Controller/2(ETCReq)  "<Throttle>"
  Control/1 -> Bus Selector5/1, Bus Creator3/2, Bus Selector4/1, Bus Selector2/1  "Controller"
  Bus Selector1/3 -> Injector Characterization/5(SysVolt09Idx), Spark Coil Characterization/2(SysVolt09Idx)  "<SysVolt09Idx>"
  Bus Selector1/4 -> Spark Advance to Start Angle/2(RPM), Injector Characterization/6(RPM)  "<RPM>"
  Bus Selector5/3 -> Injector Characterization/1(FPC [mg])  "<FPC>"
  Spark Advance to Start Angle/1(Start Angle [degCA]) -> Bus Creator1/3  "SparkStartAngle"
  Spark Coil Characterization/1(SparkDwell [ms]) -> Spark Advance to Start Angle/3(PulseWidth [ms])  "SparkDwell"
  Bus Selector1/2 -> Injector Characterization/4(MAP [kPa])  "<MAP_Angular>"
  Bus Selector1/1 -> Injector Characterization/3(BaroPress [kPa])  "<Baro>"
  Injector Characterization/1(MPW [ms]) -> Bus Creator1/1  "InjMPW"
  Bus Selector5/1 -> Spark Advance to Start Angle/1(Stop Angle [degCA])  "<SparkAdv>"
  Bus Selector5/2 -> Spark Coil Characterization/1(SparkEnergy [mJ])  "<SparkEnergy>"
  Spark Coil Characterization/2(SparkEnable [bool]) -> Bus Creator1/4  "SparkEnable"
  Injector Characterization/2(EPWOffset [ms]) -> Bus Creator1/2  "InjEPWOffset"
  SequenceCutPattern/1(Spark) -> Bus Creator1/6  "SparkCutSeq"
  SequenceCutPattern/2(Fuel) -> Bus Creator1/7  "FuelCutSeq"
  Bus Creator3/1 -> Actuators/1  "Engine"
  Bus Creator1/1 -> Bus Creator3/4  "ActuatorCharacterization"
  Diagnsotics/1 -> Bus Creator3/1  "Diagnsotics"
  Bus Selector5/4 -> Injector Characterization/2(FuelMult)  "<FuelMult>"
  motohawk_trigger/1 -> Spark Advance to Start Angle/Trigger
  motohawk_trigger1/1 -> Spark Coil Characterization/Trigger
  motohawk_data_read/1 -> CompareTo/1
  CompareTo/1 -> Electronic Throttle Controller/Enable
  Electronic Throttle Controller/2(ETC_TestActive) -> Bus Creator1/8  "ETC_TestActive"
  EGR Characterization/1(EGR) -> Bus Creator1/9  "EGR"
  Bus Selector2/1 -> EGR Characterization/1(EGR% Request)  "<EGR>"
  Bus Selector6/1 -> EGR Characterization/2(EGR% Pos)  "<EGRPos>"

== system BaseEngineController_A02_bak/Foreground/Control/Controller ==
  Sensors : Inport
  Bus Selector : BusSelector  OutputSignals=RawProcessed.EngineState
  Crank : Subsystem  TreatAsAtomicUnit=on
      mask Display: disp('CRANK STATE\nALGORITHM');
  Crank State : motohawk_control_primitives_lib/CompareTo  SystemSampleTime=-1  op===  val=2
  Documentation_Calibrations_Probes_Block : AutoDoc_lib/Documentation_Calibrations_Probes_Block
  Documentation_Left_Border : AutoDoc_lib/Documentation_Left_Border  layout=Portrait
  Documentation_Right_Border : AutoDoc_lib/Documentation_Right_Border  layout=Portrait
  Documentation_Title_Block : AutoDoc_lib/Documentation_Title_Block
  Hybrid : Subsystem
  Merge : Subsystem
  Run : Subsystem  TreatAsAtomicUnit=on
      mask Display: disp('RUN STATE\nALGORITHM');
  Run State : motohawk_control_primitives_lib/CompareTo  SystemSampleTime=-1  op===  val=3
  Stall : Subsystem  TreatAsAtomicUnit=on
      mask Display: disp('STALL STATE\nALGORITHM');
  Stall State : motohawk_control_primitives_lib/CompareTo  SystemSampleTime=-1  op===  val=1
  Actuators : Outport
  wires:
  Sensors/1 -> Bus Selector/1, Crank/1(Inputs), Run/1(Inputs), Hybrid/1(In1)
  Stall State/1 -> Stall/Enable
  Crank State/1 -> Crank/Enable
  Run State/1 -> Run/Enable
  Stall/1(Actuators) -> Merge/1(Stall)
  Run/1(Actuators) -> Merge/3(Run)
  Crank/1(Actuators) -> Merge/2(Crank)
  Bus Selector/1 -> Stall State/1, Run State/1, Crank State/1  "<EngineState>"
  Merge/1(Actuators) -> Actuators/1
  Hybrid/1(Out1) -> Merge/4(Hybrid)
